simulink model "PrkgClimaEveMgr"  (R2022b)
settings: Solver=FixedStepDiscrete  StartTime=0.0  StopTime=10.0  FixedStep=0.2  RelTol=1e-3
(part 2 of 2)

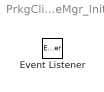
== system PrkgClimaEveMgr/PrkgClimaEveMgr_Init ==
  Event Listener : EventListener  EventName=reset

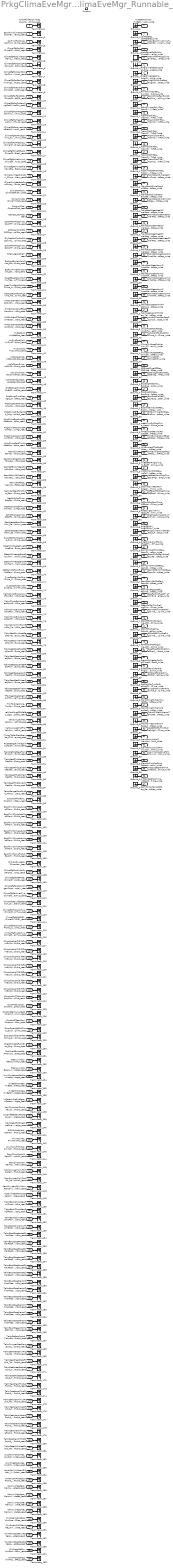
== system PrkgClimaEveMgr/PrkgClimaEveMgr_Runnable_sys ==
  AmbTFildForClimaNgcc_AmbTFildForClima_read : Inport
  BookTrvlClimaActvReqfromExsrc_BookTrvlClimaActvReqfromExsrc_read : Inport  Port=2
  CarTiIntForClima_CarTiIntForClima_read : Inport  Port=3
  ClimaCfgForCds1_ClimaCfgForCds1_read : Inport  Port=4
  ClimaCfgForClimaCtrlMan1_ClimaCfgForClimaCtrlMan1_read : Inport  Port=5
  ClimaCfgForCoolgPreCdn1_ClimaCfgForCoolgPreCdn1_read : Inport  Port=6
  ClimaCfgForCooltCircForClima1_ClimaCfgForCooltCircForClima1_read : Inport  Port=7
  ClimaCfgForEcuCooltVlvOutp1_ClimaCfgForEcuCooltVlvOutp1_read : Inport  Port=8
  ClimaCfgForExtVentnClimaCtrl_ClimaCfgForExtVentnClimaCtrl_read : Inport  Port=9
  ClimaCfgForFoldCSD_ClimaCfgForFoldCSD_read : Inport  Port=10
  ClimaCfgForForRemEngStrtPreCdn_ClimaCfgForForRemEngStrtPreCdn_read : Inport  Port=11
  ClimaCfgForHeatgPreCdnAvl1_ClimaCfgForHeatgPreCdnAvl1_read : Inport  Port=12
  ClimaCfgForHeatrTyp1_ClimaCfgForHeatrTyp1_read : Inport  Port=13
  ClimaCfgForHvacHeatrElecFrntPrsnt1_ClimaCfgForHvacHeatrElecFrntPrsnt1_read : Inport  Port=14
  ClimaCfgForPostCdnAvl1_ClimaCfgForPostCdnAvl1_read : Inport  Port=15
  ClimaCfgForPreClng1_ClimaCfgForPreClng1_read : Inport  Port=16
  ClimaCfgForVehElec1_ClimaCfgForVehElec1_read : Inport  Port=17
  ClimaCfgForVehHyb1_ClimaCfgForVehHyb1_read : Inport  Port=18
  ClimaCfgForVrntOfVehSysElec1_ClimaCfgForVrntOfVehSysElec1_read : Inport  Port=19
  ClimaOvrHeatProWarn_ClimaOvrHeatProWarn_read : Inport  Port=20
  ClimaOvrHeatReqFromExrsc_ClimaOvrHeatReqFromExrsc_read : Inport  Port=21
  ClimaStrtTiDly_ClimaStrtTiDly_read : Inport  Port=22
  ClimaTmrSts_ClimaTmrSts_read : Inport  Port=23
  CmptmtClsd_CmptmtClsd_read : Inport  Port=24
  COHSts_COHSts_read : Inport  Port=25
  CooltTForPrkgClima_CooltTForPrkgClima_read : Inport  Port=26
  DCChrgnHndlSts_DCChrgnHndlSts_read : Inport  Port=27
  DlyReqForTmrForPrkgClima_DlyReqForTmrForPrkgClima_read : Inport  Port=28
  DshbTForPrkgClima_DshbTForPrkgClima_read : Inport  Port=29
  EngT_Ngcc_EngT_read : Inport  Port=30
  ErsCmdByKeyFromExsrc_ErsCmdByKeyFromExsrc_read : Inport  Port=31
  ErsRunTimeFromExtSrc_ErsRunTimeFromExtSrc_read : Inport  Port=32
  HeatrElecNotAvlForTmr_HeatrElecNotAvlForTmr_read : Inport  Port=33
  HeatrTypSetgForPrkgClima_HeatrTypSetgForPrkgClima_read : Inport  Port=34
  HeatUpRqrdForPrkgClima_HeatUpRqrdForPrkgClima_read : Inport  Port=35
  HMIExtVentFanVolReqFromExtSrc_HMIExtVentFanVolReqFromExtSrc_read : Inport  Port=36
  HMIExtVentonoffReqFromExtSrc_HMIExtVentonoffReqFromExtSrc_read : Inport  Port=37
  HMIExtVentTSpReqFromExtSrc_HMIExtVentTSpReqFromExtSrc_read : Inport  Port=38
  HMIExtVentTStsReqFromExtSrc_HMIExtVentTStsReqFromExtSrc_read : Inport  Port=39
  HvBattSoc_HvBattSoc_read : Inport  Port=40
  HvSysCnctWoU_HvSysCnctWoU_read : Inport  Port=41
  HvSysWoU_HvSysWoU_read : Inport  Port=42
  IntCo2ActvSts_IntCo2ActvSts_read : Inport  Port=43
  IntCo2fromExsrc_IntCo2fromExsrc_read : Inport  Port=44
  KeyClimaActv_KeyClimaActv_read : Inport  Port=45
  LockgCenSts_Ngcc_LockgCenSts_read : Inport  Port=46
  OnBdChrgrHndlSts_OnBdChrgrHndlSts_read : Inport  Port=47
  PostDrvgClimaReq_Ngcc_PostDrvgClimaReq_read : Inport  Port=48
  PreClngRqrdFromExtSrc_PreClngRqrdFromExtSrc_read : Inport  Port=49
  PrkgClimaDiByCarMod_PrkgClimaDiByCarMod_read : Inport  Port=50
  PrkgClimaRqrdImdtForBattULo_PrkgClimaRqrdImdtForBattULo_read : Inport  Port=51
  PrkgClimaRqrdImdtFromActvMgr_PrkgClimaRqrdImdtFromActvMgr_read : Inport  Port=52
  PrkgClimaRqrdImdtFromExtSrc_PrkgClimaRqrdImdtFromExtSrc_read : Inport  Port=53
  PrkgClimaRqrdTmrForBattULo_PrkgClimaRqrdTmrForBattULo_read : Inport  Port=54
  RemClimaFnshd_RemClimaFnshd_read : Inport  Port=55
  RemClimaReqFromExtSrc_RemClimaReqFromExtSrc_read : Inport  Port=56
  RemStrtExtnTiReqFromExtSrc_RemStrtExtnTiReqFromExtSrc_read : Inport  Port=57
  RemStrtHvCtrlReqFromExtSrc_RemStrtHvCtrlReqFromExtSrc_read : Inport  Port=58
  RemVentFbSts_RemVentFbSts_read : Inport  Port=59
  RemVentReqfromExsrc_RemVentReqfromExsrc_read : Inport  Port=60
  ReqForPrkgClima_ReqForPrkgClima_read : Inport  Port=61
  ReqForSwtHeatrTypFromActvMgr_ReqForSwtHeatrTypFromActvMgr_read : Inport  Port=62
  RtSdlForPrkgClima_RtSdlForPrkgClima_read : Inport  Port=63
  SeatHeatgEnadFromHmi_SeatHeatgEnadFromHmi_read : Inport  Port=64
  SeatHeatgEnadFromTelm_SeatHeatgEnadFromTelm_read : Inport  Port=65
  SnsrQfOkForPrkgClima_SnsrQfOkForPrkgClima_read : Inport  Port=66
  SteerWhlHeatgDurgClimaEnadFromExsrc_SteerWhlHeatgDurgClimaEnadFromExsrc_read : Inport  Port=67
  SteerWhlHeatgEnadFromHmi_SteerWhlHeatgEnadFromHmi_read : Inport  Port=68
  SteerWhlHeatgEnadFromTelm_SteerWhlHeatgEnadFromTelm_read : Inport  Port=69
  StrtRemStsFromExsrc_StrtRemStsFromExsrc_read : Inport  Port=70
  SuspStrtActvForPrkgClima_SuspStrtActvForPrkgClima_read : Inport  Port=71
  SysDogSlpCountTi_SysDogSlpCountTi_read : Inport  Port=72
  TelmClimaExtVentnLvlFromExtSrc_TelmClimaExtVentnLvlFromExtSrc_read : Inport  Port=73
  TelmClimaExtVentnReqFromExtSrc_TelmClimaExtVentnReqFromExtSrc_read : Inport  Port=74
  TelmClimaExtVentnTempRangeFromExtSrc_TelmClimaExtVentnTempRangeFromExtSrc_read : Inport  Port=75
  TelmClimaExtVentnTSpSpclFromExtSrc_TelmClimaExtVentnTSpSpclFromExtSrc_read : Inport  Port=76
  TelmClimaTmrFromExtSrc_TelmClimaTmrFromExtSrc_read : Inport  Port=77
  TelmSeatDrvrHeatReqFromExsrc_TelmSeatDrvrHeatReqFromExsrc_read : Inport  Port=78
  TelmSeatDrvVentnReqFromExsrc_TelmSeatDrvVentnReqFromExsrc_read : Inport  Port=79
  TelmSeatPassHeatReqFromExsrc_TelmSeatPassHeatReqFromExsrc_read : Inport  Port=80
  TelmSeatPassVentnReqFromExsrc_TelmSeatPassVentnReqFromExsrc_read : Inport  Port=81
  TelmSeatSecLeHeatReqFromExsrc_TelmSeatSecLeHeatReqFromExsrc_read : Inport  Port=82
  TelmSeatSecLeVentnReqFromExsrc_TelmSeatSecLeVentnReqFromExsrc_read : Inport  Port=83
  TelmSeatSecRiHeatReqFromExsrc_TelmSeatSecRiHeatReqFromExsrc_read : Inport  Port=84
  TelmSeatSecRiVentnReqFromExsrc_TelmSeatSecRiVentnReqFromExsrc_read : Inport  Port=85
  TForHeatrPreCdngTyp_TForHeatrPreCdngTyp_read : Inport  Port=86
  TmrForPrkgClima_TmrForPrkgClima_read : Inport  Port=87
  VehModMngtGlbSafe1_Ngcc_VehModMngtGlbSafe1_read : Inport  Port=88
  VehSunLoadTot_VehSunLoadTot_read : Inport  Port=89
  VehSunLoadTotFild_VehSunLoadTotFild_read : Inport  Port=90
  ClimaCfgForStatrtDevice_ClimaCfgForStartDevice_read : Inport  Port=91
  PostHeatgRunTiEstimd_PostHeatgRunTiEstimd_read : Inport  Port=92
  TelmDefrostReqFromExsrc_TelmDefrostReqFromExsrc_read : Inport  Port=93
  TelmSeatThrdLeHeatReqFromExsrc_TelmSeatThrdLeHeatReqFromExsrc_read : Inport  Port=94
  TelmSeatThrdLeVentnReqFromExsrc_TelmSeatThrdLeVentnReqFromExsrc_read : Inport  Port=95
  TelmSeatThrdRiHeatReqFromExsrc_TelmSeatThrdRiHeatReqFromExsrc_read : Inport  Port=96
  TelmSeatThrdRiVentnReqFromExsrc_TelmSeatThrdRiVentnReqFromExsrc_read : Inport  Port=97
  TempRangeFromExtSrc_TempRangeFromExtSrc_read : Inport  Port=98
  CmptmtTFrntRaw_CmptmtTFrntRaw_read : Inport  Port=99
  BookTrvlClimaActvReq2FromExSrc_BookTrvlClimaActvReq2FromExSrc_read : Inport  Port=100
  BookTrvlClimaActvReq3FromExSrc_BookTrvlClimaActvReq3FromExSrc_read : Inport  Port=101
  BookTrvlClimaActvReq4FromExSrc_BookTrvlClimaActvReq4FromExSrc_read : Inport  Port=102
  BookTrvlClimaActvReq5FromExSrc_BookTrvlClimaActvReq5FromExSrc_read : Inport  Port=103
  BookTrvlClimaActvReq6FromExSrc_BookTrvlClimaActvReq6FromExSrc_read : Inport  Port=104
  BookTrvlClimaActvReq7FromExSrc_BookTrvlClimaActvReq7FromExSrc_read : Inport  Port=105
  BookTrvlClimaFnshd_BookTrvlClimaFnshd_read : Inport  Port=106
  ClimaActv_Ngcc_ClimaActv_read : Inport  Port=107
  ClimaCfgForAirMtrlSnsrPrsnt1_ClimaCfgForAirMtrlSnsrPrsnt1_read : Inport  Port=108
  ClimaCfgForBRAND_ClimaCfgForBRAND_read : Inport  Port=109
  ClimaCfgForCabinOxgenSupply_ClimaCfgForCabinOxgenSupply_read : Inport  Port=110
  ClimaCfgForHvacTyp_ClimaCfgForHvacTyp_read : Inport  Port=111
  ClimaCfgForLifeDetection_ClimaCfgForLifeDetection_read : Inport  Port=112
  ClimaCfgForPrpsnTyp_ClimaCfgForPrpsnTyp_read : Inport  Port=113
  ClimaCfgForRFKEY_ClimaCfgForRFKEY_read : Inport  Port=114
  ClimaCfgForSnsrHumPrsnt1_ClimaCfgForSnsrHumPrsnt1_read : Inport  Port=115
  ClimaCfgForVehTyp_ClimaCfgForVehTyp_read : Inport  Port=116
  ClimaWakUpTiDif1FromExSrc_ClimaWakUpTiDif1FromExSrc_read : Inport  Port=117
  ClimaWakUpTiDif2FromExSrc_ClimaWakUpTiDif2FromExSrc_read : Inport  Port=118
  ClimaWakUpTiDif3FromExSrc_ClimaWakUpTiDif3FromExSrc_read : Inport  Port=119
  ClimaWakUpTiDif4FromExSrc_ClimaWakUpTiDif4FromExSrc_read : Inport  Port=120
  ClimaWakUpTiDif5FromExSrc_ClimaWakUpTiDif5FromExSrc_read : Inport  Port=121
  ClimaWakUpTiDif6FromExSrc_ClimaWakUpTiDif6FromExSrc_read : Inport  Port=122
  ClimaWakUpTiDif7FromExSrc_ClimaWakUpTiDif7FromExSrc_read : Inport  Port=123
  CmptmtAirTEstimdAtRowFirst_CmptmtAirTEstimdAtRowFirst_read : Inport  Port=124
  CmptmtClngActv_CmptmtClngActv_read : Inport  Port=125
  CmptmtRelHum_Ngcc_CmptmtRelHum_read : Inport  Port=126
  CmptmtTRearRaw_CmptmtTRearRaw_read : Inport  Port=127
  DoorOpenStsForClima_DoorOpenStsForClima_read : Inport  Port=128
  DoorStsWithOutTrForClima_DoorStsWithOutTrForClima_read : Inport  Port=129
  EngSt1WdStsFromExsrc_EngSt1WdStsFromExsrc_read : Inport  Port=130
  FrntHvacBlowerSts_FrntHvacBlowerSts_read : Inport  Port=131
  GearLvrIndcn_GearLvrIndcn_read : Inport  Port=132
  GearLvrIndcn_GearLvrIndcn_IsUpdated_read : Inport  Port=133
  HmiWinterLockCarPrevnIceSetNgcc_HmiWinterLockCarPrevnIceSetNgcc_read : Inport  Port=134
  HvBattMntnReq_HvBattMntnReq_read : Inport  Port=135
  HvBattMntnReq_HvBattMntnReq_IsUpdated_read : Inport  Port=136
  InflateAirSrcSwtNgcc_InflateAirSrcSwtNgcc_read : Inport  Port=137
  KeyClimaActvFnshd_KeyClimaActvFnshd_read : Inport  Port=138
  LonglifeBatteryReqNgcc_LonglifeBatteryReqNgcc_read : Inport  Port=139
  MemReadForPrkgClimaEveMgr_MemReadForPrkgClimaEveMgr_read : Inport  Port=140
  OGMAirSrcFnshd_OGMAirSrcFnshd_read : Inport  Port=141
  PrvntWinFrz_PrvntWinFrz_read : Inport  Port=142
  PrvntWinFrzFnshd_PrvntWinFrzFnshd_read : Inport  Port=143
  RemClimaFnshd1_RemClimaFnshd1_read : Inport  Port=144
  RemClimaWarn_RemClimaWarn_read : Inport  Port=145
  RemOxygenSplyFnshd_RemOxygenSplyFnshd_read : Inport  Port=146
  RemOxygenSplyFnshd1_RemOxygenSplyFnshd1_read : Inport  Port=147
  RemOxygenSplyWarn_RemOxygenSplyWarn_read : Inport  Port=148
  TcsOvrheatdProtnStsNgcc_TcsOvrheatdProtnStsNgcc_read : Inport  Port=149
  TelmBookClimaReqHmiCmptmtTSpSpclFromExtSrc_TelmBookClimaReqHmiCmptmtTSpSpclFromExtSrc_read : Inport  Port=150
  TelmBookClimaReqOnOffNoReqFromExtSrc_TelmBookClimaReqOnOffNoReqFromExtSrc_read : Inport  Port=151
  TelmBookClimaReqTempRangeFromExtSrc_TelmBookClimaReqTempRangeFromExtSrc_read : Inport  Port=152
  TelmBookClimaTmrFromExtSrc_TelmBookClimaTmrFromExtSrc_read : Inport  Port=153
  TelmBookSeatHeatClimalReqDrvHeatClimaLvlFromExtSrc_TelmBookSeatHeatClimalReqDrvHeatClimaLvlFromExtSrc_read : Inport  Port=154
  TelmBookSeatHeatClimalReqPassHeatClimaLvlFromExtSrc_TelmBookSeatHeatClimalReqPassHeatClimaLvlFromExtSrc_read : Inport  Port=155
  TelmBookSeatHeatClimalReqSecLeHeatClimaLvlFromExtSrc_TelmBookSeatHeatClimalReqSecLeHeatClimaLvlFromExtSrc_read : Inport  Port=156
  TelmBookSeatHeatClimalReqSecRiHeatClimaLvlFromExtSrc_TelmBookSeatHeatClimalReqSecRiHeatClimaLvlFromExtSrc_read : Inport  Port=157
  TelmBookSeatHeatClimalReqThrdLeHeatClimaLvlFromExtSrc_TelmBookSeatHeatClimalReqThrdLeHeatClimaLvlFromExtSrc_read : Inport  Port=158
  TelmBookSeatHeatClimalReqThrdRiHeatClimaLvlFromExtSrc_TelmBookSeatHeatClimalReqThrdRiHeatClimaLvlFromExtSrc_read : Inport  Port=159
  TelmBookSeatVentnClimalReqDrvVentnClimaLvlFromExtSrc_TelmBookSeatVentnClimalReqDrvVentnClimaLvlFromExtSrc_read : Inport  Port=160
  TelmBookSeatVentnClimalReqPassVentnClimaLvlFromExtSrc_TelmBookSeatVentnClimalReqPassVentnClimaLvlFromExtSrc_read : Inport  Port=161
  TelmBookSeatVentnClimalReqSecLeVentnClimaLvlFromExtSrc_TelmBookSeatVentnClimalReqSecLeVentnClimaLvlFromExtSrc_read : Inport  Port=162
  TelmBookSeatVentnClimalReqSecRiVentnClimaLvlFromExtSrc_TelmBookSeatVentnClimalReqSecRiVentnClimaLvlFromExtSrc_read : Inport  Port=163
  TelmBookSeatVentnClimalReqThrdLeVentnClimaLvlFromExtSrc_TelmBookSeatVentnClimalReqThrdLeVentnClimaLvlFromExtSrc_read : Inport  Port=164
  TelmBookSeatVentnClimalReqThrdRiVentnClimaLvlFromExtSrc_TelmBookSeatVentnClimalReqThrdRiVentnClimaLvlFromExtSrc_read : Inport  Port=165
  TelmBookSteerWhlHeatClimalReqFromExtSrc_TelmBookSteerWhlHeatClimalReqFromExtSrc_read : Inport  Port=166
  TelmDefrostFnshd_TelmDefrostFnshd_read : Inport  Port=167
  TelmOxygenReqFromExSrc_TelmOxygenReqFromExSrc_read : Inport  Port=168
  TelmSeatHeatDrvrFnshd_TelmSeatHeatDrvrFnshd_read : Inport  Port=169
  TelmSeatHeatPassFnshd_TelmSeatHeatPassFnshd_read : Inport  Port=170
  TelmSeatHeatSecLeFnshd_TelmSeatHeatSecLeFnshd_read : Inport  Port=171
  TelmSeatHeatSecRiFnshd_TelmSeatHeatSecRiFnshd_read : Inport  Port=172
  TelmSeatHeatThrdLeFnshd_TelmSeatHeatThrdLeFnshd_read : Inport  Port=173
  TelmSeatHeatThrdRiFnshd_TelmSeatHeatThrdRiFnshd_read : Inport  Port=174
  TelmSeatVentnDrvrFnshd_TelmSeatVentnDrvrFnshd_read : Inport  Port=175
  TelmSeatVentnPassFnshd_TelmSeatVentnPassFnshd_read : Inport  Port=176
  TelmSeatVentnSecLeFnshd_TelmSeatVentnSecLeFnshd_read : Inport  Port=177
  TelmSeatVentnSecRiFnshd_TelmSeatVentnSecRiFnshd_read : Inport  Port=178
  TelmSeatVentnThrdLeFnshd_TelmSeatVentnThrdLeFnshd_read : Inport  Port=179
  TelmSeatVentnThrdRiFnshd_TelmSeatVentnThrdRiFnshd_read : Inport  Port=180
  TelmSteerWhlHeatFnshd_TelmSteerWhlHeatFnshd_read : Inport  Port=181
  UnLckClimaActvReq_UnLckClimaActvReq_read : Inport  Port=182
  UnLckHeatActvReq_UnLckHeatActvReq_read : Inport  Port=183
  VentnForWinOpenGlbActv_VentnForWinOpenGlbActv_read : Inport  Port=184
  VTMOverTProtectionFnshd_VTMOverTProtectionFnshd_read : Inport  Port=185
  WarnLvlMsgNgcc_WarnLvlMsgNgcc_read : Inport  Port=186
  WarnLvlMsgNgcc_WarnLvlMsgNgcc_IsUpdated_read : Inport  Port=187
  WarnLvlMsgUWB_WarnLvlMsgUWB_read : Inport  Port=188
  WarnLvlMsgUWB_WarnLvlMsgUWB_IsUpdated_read : Inport  Port=189
  WinOpenLDACReq_WinOpenLDACReq_read : Inport  Port=190
  WinOpenLDACReqUWB_WinOpenLDACReqUWB_read : Inport  Port=191
  WinPosnStsAtDrvrReNgcc_WinPosnStsAtDrvrReNgcc_read : Inport  Port=192
  WinPosnStsAtPassReNgcc_WinPosnStsAtPassRe_read : Inport  Port=193
  WinPosnStsDrv_WinPosnStsAtDrv_read : Inport  Port=194
  WinPosnStsPass_WinPosnStsAtPass_read : Inport  Port=195
  function : TriggerPort  TriggerType=function-call  VariantControl=(inherit)  SampleTimeType=periodic  SampleTime=-1
  Ground_{1..4,6..97} (96 alike) : Ground
  Ground_5 : Subsystem
  Terminator_{1..195} (195 alike) : Terminator
  HvBattMntnActv_HvBattMntnActv_write : Outport
      settings: EnsureOutportIsVirtual=on
  LDACState_LDACState_write : Outport  Port=2
      settings: EnsureOutportIsVirtual=on
  MemBookTrvlClimaTi_MemBookTrvlClimaTi_write : Outport  Port=3
      settings: EnsureOutportIsVirtual=on
  MemSaveFlgForPrkgClimaEveMgr_MemSaveFlgForPrkgClimaEveMgr_write : Outport  Port=4
      settings: EnsureOutportIsVirtual=on
  MemWrForPrkgClimaEveMgr_MemWrForPrkgClimaEveMgr_write : Outport  Port=5
      settings: EnsureOutportIsVirtual=on
  PrkgClimaReqExcpSeat_PrkgClimaReqExcpSeat_write : Outport  Port=6
      settings: EnsureOutportIsVirtual=on
  PrkgClimaReqPrio_PrkgClimaReqPrio_write : Outport  Port=7
      settings: EnsureOutportIsVirtual=on
  PrkgOGMAirSrcReq_PrkgOGMAirSrcReq_write : Outport  Port=8
      settings: EnsureOutportIsVirtual=on
  PrvntWinActvReq_PrvntWinActvReq_write : Outport  Port=9
      settings: EnsureOutportIsVirtual=on
  RemClimaEgyExsrcReqPlus_RemClimaEgyExsrcReqPlus_write : Outport  Port=10
      settings: EnsureOutportIsVirtual=on
  RemOxygenSplyReq_RemOxygenSplyReq_write : Outport  Port=11
      settings: EnsureOutportIsVirtual=on
  TcamClcltTiFb1_TcamClcltTiFb1_write : Outport  Port=12
      settings: EnsureOutportIsVirtual=on
  TcamClcltTiFb2_TcamClcltTiFb2_write : Outport  Port=13
      settings: EnsureOutportIsVirtual=on
  TcamClcltTiFb3_TcamClcltTiFb3_write : Outport  Port=14
      settings: EnsureOutportIsVirtual=on
  TcamClcltTiFb4_TcamClcltTiFb4_write : Outport  Port=15
      settings: EnsureOutportIsVirtual=on
  TcamClcltTiFb5_TcamClcltTiFb5_write : Outport  Port=16
      settings: EnsureOutportIsVirtual=on
  TcamClcltTiFb6_TcamClcltTiFb6_write : Outport  Port=17
      settings: EnsureOutportIsVirtual=on
  TcamClcltTiFb7_TcamClcltTiFb7_write : Outport  Port=18
      settings: EnsureOutportIsVirtual=on
  TcsOvrheatdProtnReq_TcsOvrheatdProtnReq_write : Outport  Port=19
      settings: EnsureOutportIsVirtual=on
  TelmBookClimaReqHmiCmptmtTSpSpclStrtReq_TelmBookClimaReqHmiCmptmtTSpSpclStrtReq_write : Outport  Port=20
      settings: EnsureOutportIsVirtual=on
  TelmBookClimaReqOnOffNoReqStrtReq_TelmBookClimaReqOnOffNoReqStrtReq_write : Outport  Port=21
      settings: EnsureOutportIsVirtual=on
  TelmBookClimaReqTempRangeStrtReq_TelmBookClimaReqTempRangeStrtReq_write : Outport  Port=22
      settings: EnsureOutportIsVirtual=on
  TelmBookClimaTmrStrtReq_TelmBookClimaTmrStrtReq_write : Outport  Port=23
      settings: EnsureOutportIsVirtual=on
  TelmBookSeatHeatClimalReqDrvHeatClimaLvlStrtReq_TelmBookSeatHeatClimalReqDrvHeatClimaLvlStrtReq_write : Outport  Port=24
      settings: EnsureOutportIsVirtual=on
  TelmBookSeatHeatClimalReqPassHeatClimaLvlStrtReq_TelmBookSeatHeatClimalReqPassHeatClimaLvlStrtReq_write : Outport  Port=25
      settings: EnsureOutportIsVirtual=on
  TelmBookSeatHeatClimalReqSecLeHeatClimaLvlStrtReq_TelmBookSeatHeatClimalReqSecLeHeatClimaLvlStrtReq_write : Outport  Port=26
      settings: EnsureOutportIsVirtual=on
  TelmBookSeatHeatClimalReqSecRiHeatClimaLvlStrtReq_TelmBookSeatHeatClimalReqSecRiHeatClimaLvlStrtReq_write : Outport  Port=27
      settings: EnsureOutportIsVirtual=on
  TelmBookSeatHeatClimalReqThrdLeHeatClimaLvlStrtReq_TelmBookSeatHeatClimalReqThrdLeHeatClimaLvlStrtReq_write : Outport  Port=28
      settings: EnsureOutportIsVirtual=on
  TelmBookSeatHeatClimalReqThrdRiHeatClimaLvlStrtReq_TelmBookSeatHeatClimalReqThrdRiHeatClimaLvlStrtReq_write : Outport  Port=29
      settings: EnsureOutportIsVirtual=on
  TelmBookSeatVentnClimalReqDrvVentnClimaLvlStrtReq_TelmBookSeatVentnClimalReqDrvVentnClimaLvlStrtReq_write : Outport  Port=30
      settings: EnsureOutportIsVirtual=on
  TelmBookSeatVentnClimalReqPassVentnClimaLvlStrtReq_TelmBookSeatVentnClimalReqPassVentnClimaLvlStrtReq_write : Outport  Port=31
      settings: EnsureOutportIsVirtual=on
  TelmBookSeatVentnClimalReqSecLeVentnClimaLvlStrtReq_TelmBookSeatVentnClimalReqSecLeVentnClimaLvlStrtReq_write : Outport  Port=32
      settings: EnsureOutportIsVirtual=on
  TelmBookSeatVentnClimalReqSecRiVentnClimaLvlStrtReq_TelmBookSeatVentnClimalReqSecRiVentnClimaLvlStrtReq_write : Outport  Port=33
      settings: EnsureOutportIsVirtual=on
  TelmBookSeatVentnClimalReqThrdLeVentnClimaLvlStrtReq_TelmBookSeatVentnClimalReqThrdLeVentnClimaLvlStrtReq_write : Outport  Port=34
      settings: EnsureOutportIsVirtual=on
  TelmBookSeatVentnClimalReqThrdRiVentnClimaLvlStrtReq_TelmBookSeatVentnClimalReqThrdRiVentnClimaLvlStrtReq_write : Outport  Port=35
      settings: EnsureOutportIsVirtual=on
  TelmBookSteerWhlHeatClimalReqStrtReq_TelmBookSteerWhlHeatClimalReqStrtReq_write : Outport  Port=36
      settings: EnsureOutportIsVirtual=on
  TelmSeatVentnThrdReq_TelmSeatVentnThrdReq_write : Outport  Port=37
      settings: EnsureOutportIsVirtual=on
  UnLckClimaActvReqSt_UnLckClimaActvReqSt_write : Outport  Port=38
      settings: EnsureOutportIsVirtual=on
  UnLckHeatActvReqSt_UnLckHeatActvReqSt_write : Outport  Port=39
      settings: EnsureOutportIsVirtual=on
  ActvRqrdForPostPrkgClima_ActvRqrdForPostPrkgClima_write : Outport  Port=40
      settings: EnsureOutportIsVirtual=on
  ClimaOvrHeatProWarnIni_ClimaOvrHeatProWarnIni_write : Outport  Port=41
      settings: EnsureOutportIsVirtual=on
  ClimaOvrHeatStrtReq_ClimaOvrHeatStrtReq_write : Outport  Port=42
      settings: EnsureOutportIsVirtual=on
  ClimaStrtTi_ClimaStrtTi_write : Outport  Port=43
      settings: EnsureOutportIsVirtual=on
  CSDUsgChgeOffReq_CSDUsgChgeOffReq_write : Outport  Port=44
      settings: EnsureOutportIsVirtual=on
  CSDUsgChgeOnReq_CSDUsgChgeOnReq_write : Outport  Port=45
      settings: EnsureOutportIsVirtual=on
  EcuDogKeepActv_EcuDogKeepActv_write : Outport  Port=46
      settings: EnsureOutportIsVirtual=on
  EcuDogSlpReq_EcuDogSlpReq_write : Outport  Port=47
      settings: EnsureOutportIsVirtual=on
  EcuDogSlpSetTi_EcuDogSlpSetTi_write : Outport  Port=48
      settings: EnsureOutportIsVirtual=on
  ErsCmdByKeyStrtReq_ErsCmdByKeyStrtReq_write : Outport  Port=49
      settings: EnsureOutportIsVirtual=on
  ErsRunTimelStrtReq_ErsRunTimelStrtReq_write : Outport  Port=50
      settings: EnsureOutportIsVirtual=on
  HeatrTypForPrkgClima_HeatrTypForPrkgClima_write : Outport  Port=51
      settings: EnsureOutportIsVirtual=on
  HMIExtVentFanVolReqStrtReq_HMIExtVentFanVolReqStrtReq_write : Outport  Port=52
      settings: EnsureOutportIsVirtual=on
  HMIExtVentonoffReqStrtReq_HMIExtVentonoffReqStrtReq_write : Outport  Port=53
      settings: EnsureOutportIsVirtual=on
  HMIExtVentTSpReqStrtReq_HMIExtVentTSpReqStrtReq_write : Outport  Port=54
      settings: EnsureOutportIsVirtual=on
  HMIExtVentTStsReqStrtReq_HMIExtVentTStsReqStrtReq_write : Outport  Port=55
      settings: EnsureOutportIsVirtual=on
  HvBattForPrkgClima_HvBattForPrkgClima_write : Outport  Port=56
      settings: EnsureOutportIsVirtual=on
  IntCo2MontrStrtReq_IntCo2MontrStrtReq_write : Outport  Port=57
      settings: EnsureOutportIsVirtual=on
  NetReqFromPrkgClimaEveMgr_NetReqFromPrkgClimaEveMgr_write : Outport  Port=58
      settings: EnsureOutportIsVirtual=on
  ObsvrActvnReqFromPrkgClima_ObsvrActvnReqFromPrkgClima_write : Outport  Port=59
      settings: EnsureOutportIsVirtual=on
  OffRqrdForPrkgClima_OffRqrdForPrkgClima_write : Outport  Port=60
      settings: EnsureOutportIsVirtual=on
  OffRqrdForRemClima_OffRqrdForRemClima_write : Outport  Port=61
      settings: EnsureOutportIsVirtual=on
  PostDrvgClimaAvl_Ngcc_PostDrvgClimaAvl_write : Outport  Port=62
      settings: EnsureOutportIsVirtual=on
  PrePrkgClimaNotAvlForHvSysWoU_PrePrkgClimaNotAvlForHvSysWoU_write : Outport  Port=63
      settings: EnsureOutportIsVirtual=on
  PrkgCDSAvl_PrkgCDSAvl_write : Outport  Port=64
      settings: EnsureOutportIsVirtual=on
  PrkgClimaAllwdForBattULo_PrkgClimaAllwdForBattULo_write : Outport  Port=65
      settings: EnsureOutportIsVirtual=on
  PrkgClimaAllwdForSwtHeatrTyp_PrkgClimaAllwdForSwtHeatrTyp_write : Outport  Port=66
      settings: EnsureOutportIsVirtual=on
  RemClimaallExsrcReq_RemClimaallExsrcReq_write : Outport  Port=67
      settings: EnsureOutportIsVirtual=on
  RemClimaEgyExsrcReq_RemClimaEgyExsrcReq_write : Outport  Port=68
      settings: EnsureOutportIsVirtual=on
  RemClimaTmrStrtReq_RemClimaTmrStrtReq_write : Outport  Port=69
      settings: EnsureOutportIsVirtual=on
  RemStrtExtnTiStrtReq_RemStrtExtnTiStrtReq_write : Outport  Port=70
      settings: EnsureOutportIsVirtual=on
  RemStrtHvCtrlStrtReq_RemStrtHvCtrlStrtReq_write : Outport  Port=71
      settings: EnsureOutportIsVirtual=on
  RemVentReqStrtReq_RemVentReqStrtReq_write : Outport  Port=72
      settings: EnsureOutportIsVirtual=on
  ReqForSwtHeatrTypFromEveMgr_ReqForSwtHeatrTypFromEveMgr_write : Outport  Port=73
      settings: EnsureOutportIsVirtual=on
  RtBasSdlForTmrPrePrkgClima_RtBasSdlForTmrPrePrkgClima_write : Outport  Port=74
      settings: EnsureOutportIsVirtual=on
  RtCmftForImdtPrePrkgClima_RtCmftForImdtPrePrkgClima_write : Outport  Port=75
      settings: EnsureOutportIsVirtual=on
  RtCmftForPostPrkgClima_RtCmftForPostPrkgClima_write : Outport  Port=76
      settings: EnsureOutportIsVirtual=on
  RtCmftForPreClng_RtCmftForPreClng_write : Outport  Port=77
      settings: EnsureOutportIsVirtual=on
  RtCmftForTmrPrePrkgClima_RtCmftForTmrPrePrkgClima_write : Outport  Port=78